simulink model "wlan_phy_rx_pmd"  (Simulink 7.8)
settings: Solver=VariableStepDiscrete  SolverMode=SingleTasking  StartTime=0.0  StopTime=rx_sim_time  RelTol=1e-3
PostLoadFcn:
  | wlan_phy_rx_init
InitFcn:
  | wlan_phy_rx_init
StartFcn:
  | wlan_phy_rx_init
values from neighbouring files (symbols this model uses):
  barker_seq20 = 0.95 * circshift(barker_seq20, [0 4]) ./ max(abs(barker_seq20))    [wlan_phy_rx_init.m]
  bit_scrambler_lfsr_bytes = sum(bit_scrambler_lfsr_bytes .* repmat(2.^[0:7], 127, 1),2)    [wlan_phy_rx_init.m]
  deinterleave_ROM = [deinterleave_ROM interleave_11n_64QAM zeros(1, 512-length(interleave_11n_64QAM))]    [wlan_phy_rx_init.m]
  longCorr_coef_i = [3*real(longCorr_coef)]    [wlan_phy_rx_init.m]
  longCorr_coef_q = [3*imag(longCorr_coef)]    [wlan_phy_rx_init.m]
  MAX_CP_LEN = 32    [wlan_phy_rx_init.m]
  MAX_NCBPS = NCBPS_11n_64QAM    (= 312)    [wlan_phy_rx_init.m]
  MAX_NUM_BYTES = 2^16-1    (= 65535)    [wlan_phy_rx_init.m]
  MAX_NUM_SC = 64    [wlan_phy_rx_init.m]
  MAX_NUM_SYMS = 600    [wlan_phy_rx_init.m]
  NCBPS_11a_BPSK = 48    [wlan_phy_rx_init.m]
  NDBPS_11a_BPSK12 = 24    [wlan_phy_rx_init.m]
  PHY_SIGNAL_MIN_LEN = 14    [wlan_phy_rx_init.m]
  preFFT_sampBuff_numSamps = 4*MAX_NUM_SC    (= 256)    [wlan_phy_rx_init.m]
  rx_sim_sig_samp_time = 8    [wlan_phy_rx_init.m]
  scr_ind_rev = zeros(1,128)    [wlan_phy_rx_init.m]
undefined symbols (not defined in the file or in its folder): gcbh, mcs_rom_11ag, mcs_rom_11n, xlBlockLoadCallback, xlGetNormalizedPeriod, xlipgetpartsetting
libraries: xbsIndex_r4 x3862, simulink x1, xbsEDKLib_r4 x1
(part 8 of 23)

== system wlan_phy_rx_pmd/CFO & Samp Buffer/Samp Buffer/S-R Latch ==
  S : Inport
  R : Inport  Port=2
  Constant1 : xbsIndex_r4/Constant  const=1  gui_display_data_type=Fixed-point  arith_type=Boolean  n_bits=1  bin_pt=0  preci_type=Single  exp_width=8  frac_width=24  explicit_period=off  period=1  equ=P=C  opselect=C  inp2=PCIN>>17  opr=+  inp1=P  carry=CIN  dbl_ovrd=off  block_type=constant
      settings: block_version=VER_STRING_GOES_HERE
  Register2 : xbsIndex_r4/Register  init=0  rst=on  en=on  dbl_ovrd=off  block_type=register
      settings: xl_area=[1 1 0 0 0 0 0]  block_version=VER_STRING_GOES_HERE
  Q : Outport
  wires:
  Constant1/1 -> Register2/1
  R/1 -> Register2/2
  Register2/1 -> Q/1
  S/1 -> Register2/3

== system wlan_phy_rx_pmd/CFO & Samp Buffer/Samp Buffer/Slice ==
  IQ : Inport
  Reinterpret2 : xbsIndex_r4/Reinterpret  force_arith_type=on  arith_type="Signed  (2's comp)"  force_bin_pt=on  bin_pt=15  block_type=reinterpret
  Reinterpret3 : xbsIndex_r4/Reinterpret  force_arith_type=on  arith_type="Signed  (2's comp)"  force_bin_pt=on  bin_pt=15  block_type=reinterpret
  Slice : xbsIndex_r4/Slice  nbits=16  boolean_output=off  mode=Upper Bit Location + Width  bit1=0  base1=MSB of Input  bit0=0  base0=LSB of Input  dbl_ovrd=off  block_type=slice
  Slice1 : xbsIndex_r4/Slice  nbits=16  boolean_output=off  mode=Lower Bit Location + Width  bit1=0  base1=MSB of Input  bit0=0  base0=LSB of Input  dbl_ovrd=off  block_type=slice
  I : Outport
  Q : Outport  Port=2
  wires:
  Slice/1 -> Reinterpret2/1
  Slice1/1 -> Reinterpret3/1
  IQ/1 -> Slice1/1, Slice/1
  Reinterpret2/1 -> I/1
  Reinterpret3/1 -> Q/1

== system wlan_phy_rx_pmd/Chan Est & EQ/Chan Est/Chan Est Offload ==
  note: These signals route to the packet buffer interface. The
      | chan ests are written to dedicated bytes in each packet
      | buffer, ahead of the packet payload. This is way
      | better than using a Sysgen shared memory block here
      | since it allows CPU High to treat the H ests as meta data
      | alongside the packet contents, with no further
      | involvement from the PHY or CPU Low.
      |
      | Estimates are written to the pkt buf after all training is
      | complete. Training from the L-LTF are written in OFDM
      | symbol 2 (SIGNAL). For 11n Rx the estimates are over-
      | written during sybmol 7 (first DATA). This assumes 1
      | spatial stream.
      |
      | The timing of CHAN_EST_VALID is critical. This signal
      | becomes the write enable on the pkt buf BRAM interface.
      | The CHAN_EST_VALID signal must *not* assert when actual
      | bytes are being written to the pkt buf. Asserting on OFDM syms
      | 2 and 7 is safe- there cannot be any decoded bits at these symbol
      | intervals. Any changes to pkt buf or decoder timing must be
      | checked for conflicts in BRAM write enable assertion between
      | chan ests and decoded bytes!
  x_ind : Inport
  x_I : Inport  Port=2
  x_Q : Inport  Port=3
  x_valid : Inport  Port=4
  sym_ind : Inport  Port=5
  Concat : xbsIndex_r4/Concat  num_inputs=2  dbl_ovrd=off  block_type=concat
  Constant1 : xbsIndex_r4/Constant  const=7  gui_display_data_type=Fixed-point  arith_type=Unsigned  n_bits=3  bin_pt=0  preci_type=Single  exp_width=8  frac_width=24  explicit_period=off  period=8  equ=P=C  opselect=C  inp2=PCIN>>17  opr=+  inp1=P  carry=CIN  dbl_ovrd=off  block_type=constant
  Constant3 : xbsIndex_r4/Constant  const=2  gui_display_data_type=Fixed-point  arith_type=Unsigned  n_bits=2  bin_pt=0  preci_type=Single  exp_width=8  frac_width=24  explicit_period=off  period=8  equ=P=C  opselect=C  inp2=PCIN>>17  opr=+  inp1=P  carry=CIN  dbl_ovrd=off  block_type=constant
  Convert1 : xbsIndex_r4/Convert  gui_display_data_type=Fixed-point  arith_type="Signed  (2's comp)"  n_bits=16  bin_pt=15  float_type=Single  exp_bits=8  fraction_bits=24  quantization=Truncate  overflow=Wrap  en=off  latency=0  dbl_ovrd=off  pipeline=off  block_type=convert
  Convert2 : xbsIndex_r4/Convert  gui_display_data_type=Fixed-point  arith_type="Signed  (2's comp)"  n_bits=16  bin_pt=15  float_type=Single  exp_bits=8  fraction_bits=24  quantization=Truncate  overflow=Wrap  en=off  latency=0  dbl_ovrd=off  pipeline=off  block_type=convert
  Logical1 : xbsIndex_r4/Logical  logical_function=OR  inputs=2  en=off  latency=0  precision=Full  arith_type=Unsigned  n_bits=16  bin_pt=0  align_bp=on  dbl_ovrd=off  block_type=logical
  Logical3 : xbsIndex_r4/Logical  logical_function=AND  inputs=2  en=off  latency=0  precision=Full  arith_type=Unsigned  n_bits=16  bin_pt=0  align_bp=on  dbl_ovrd=off  block_type=logical
  Logical4 : xbsIndex_r4/Logical  logical_function=AND  inputs=2  en=off  latency=0  precision=Full  arith_type=Unsigned  n_bits=16  bin_pt=0  align_bp=on  dbl_ovrd=off  block_type=logical
  Reinterpret : xbsIndex_r4/Reinterpret  force_arith_type=on  arith_type=Unsigned  force_bin_pt=on  bin_pt=0  block_type=reinterpret
  Reinterpret1 : xbsIndex_r4/Reinterpret  force_arith_type=on  arith_type=Unsigned  force_bin_pt=on  bin_pt=0  block_type=reinterpret
  Relational1 : xbsIndex_r4/Relational  mode=a=b  en=off  latency=0  dbl_ovrd=off  block_type=relational
  Relational3 : xbsIndex_r4/Relational  mode=a=b  en=off  latency=0  dbl_ovrd=off  block_type=relational
  tag scope: CHAN_EST_IND=global, CHAN_EST_IQ_32b=global, CHAN_EST_VALID=global
  wires:
  x_ind/1 -> [CHAN_EST_IND]
  x_I/1 -> Convert1/1
  x_Q/1 -> Convert2/1
  x_valid/1 -> Logical3/1
  sym_ind/1 -> Relational3/2, Relational1/1
  Convert2/1 -> Reinterpret1/1
  Convert1/1 -> Reinterpret/1
  Logical3/1 -> [CHAN_EST_VALID]
  Constant3/1 -> Relational3/1
  Reinterpret1/1 -> Concat/2
  Reinterpret/1 -> Concat/1
  Concat/1 -> [CHAN_EST_IQ_32b]
  Relational3/1 -> Logical1/1
  Logical1/1 -> Logical3/2
  Logical4/1 -> Logical1/2
  Constant1/1 -> Relational1/2
  Relational1/1 -> Logical4/1
  [RX_PHY_MODE_11N] -> Logical4/2

== system wlan_phy_rx_pmd/Chan Est & EQ/Chan Est/Control ==
  note: OFDM Symbols in 11a/g:
      | 0: LTF (BPSK)
      | 1: LTF (BPSK)
      | 2: SIG (BPSK)
      | 3-N: Data
  note: OFDM Symbols in 11n SISO:
      | 0: L-LTF (BPSK)
      | 1: L-LTF (BPSK)
      | 2: L-SIG (BPSK)
      | 3: HT-SIG1 (QBPSK)
      | 4: HT-SIG2 (QBPSK)
      | 5: HT-STF
      | 6: HT-LFT1
      | 7-N: Data
  note: OFDM Symbols in 11ac SISO:
      | 0: L-LTF (BPSK)
      | 1: L-LTF (BPSK)
      | 2: L-SIG (BPSK)
      | 3: VHT-SIGA1 (BPSK)
      | 4: VHT-SIGA2 (QBPSK)
      | 5: VHT-STF
      | 6: VHT-LFT1
      | 7: VHT-SIGB
      | 8-N: Data
  note: Downstream blocks should ignore Rx I/Q and H_est I/Q
      | during all training symbols in all PHY modes
  Sym Ind : Inport
  Constant1 : xbsIndex_r4/Constant  const=2  gui_display_data_type=Fixed-point  arith_type=Unsigned  n_bits=2  bin_pt=0  preci_type=Single  exp_width=8  frac_width=24  explicit_period=off  period=8  equ=P=C  opselect=C  inp2=PCIN>>17  opr=+  inp1=P  carry=CIN  dbl_ovrd=off  block_type=constant
  Constant2 : xbsIndex_r4/Constant  const=0  gui_display_data_type=Fixed-point  arith_type=Unsigned  n_bits=2  bin_pt=0  preci_type=Single  exp_width=8  frac_width=24  explicit_period=off  period=8  equ=P=C  opselect=C  inp2=PCIN>>17  opr=+  inp1=P  carry=CIN  dbl_ovrd=off  block_type=constant
  Constant3 : xbsIndex_r4/Constant  const=5  gui_display_data_type=Fixed-point  arith_type=Unsigned  n_bits=3  bin_pt=0  preci_type=Single  exp_width=8  frac_width=24  explicit_period=off  period=8  equ=P=C  opselect=C  inp2=PCIN>>17  opr=+  inp1=P  carry=CIN  dbl_ovrd=off  block_type=constant
  Constant4 : xbsIndex_r4/Constant  const=6  gui_display_data_type=Fixed-point  arith_type=Unsigned  n_bits=3  bin_pt=0  preci_type=Single  exp_width=8  frac_width=24  explicit_period=off  period=8  equ=P=C  opselect=C  inp2=PCIN>>17  opr=+  inp1=P  carry=CIN  dbl_ovrd=off  block_type=constant
  Logical1 : xbsIndex_r4/Logical  logical_function=AND  inputs=2  en=off  latency=0  precision=Full  arith_type=Unsigned  n_bits=16  bin_pt=0  align_bp=on  dbl_ovrd=off  block_type=logical
  Logical{2..3,5,7} (4 alike) : xbsIndex_r4/Logical  logical_function=OR  inputs=2  en=off  latency=0  precision=Full  arith_type=Unsigned  n_bits=16  bin_pt=0  align_bp=on  dbl_ovrd=off  block_type=logical
  Logical4 : xbsIndex_r4/Logical  logical_function=AND  inputs=2  en=off  latency=0  precision=Full  arith_type=Unsigned  n_bits=16  bin_pt=0  align_bp=on  dbl_ovrd=off  block_type=logical
  Logical6 : xbsIndex_r4/Logical  logical_function=AND  inputs=2  en=off  latency=0  precision=Full  arith_type=Unsigned  n_bits=16  bin_pt=0  align_bp=on  dbl_ovrd=off  block_type=logical
  Relational1 : xbsIndex_r4/Relational  mode=a<b  en=off  latency=0  dbl_ovrd=off  block_type=relational
  Relational{2,4..6} (4 alike) : xbsIndex_r4/Relational  mode=a=b  en=off  latency=0  dbl_ovrd=off  block_type=relational
  Relational3 : xbsIndex_r4/Relational  mode=a>=b  en=off  latency=0  dbl_ovrd=off  block_type=relational
  Est Overwrite : Outport
  Est WrEn : Outport  Port=2
  Scale : Outport  Port=3
  Sym Invalid : Outport  Port=4
  wires:
  Constant2/1 -> Relational2/1
  Constant1/1 -> Relational1/2
  Sym Ind/1 -> Relational2/2, Relational1/1, Relational4/1, Relational3/1, Relational5/1, Relational6/1
  Relational2/1 -> Logical2/1
  Relational1/1 -> Logical3/1, Logical7/1
  Logical2/1 -> Est Overwrite/1
  Logical4/1 -> Logical3/2, Logical2/2
  Logical3/1 -> Est WrEn/1
  Relational4/1 -> Logical4/1
  Constant4/1 -> Relational4/2, Relational3/2, Relational5/2
  [RX_PHY_MODE_11N_AC] -> Logical4/2, Logical1/2, Logical6/1
  Relational3/1 -> Logical1/1
  Logical1/1 -> Scale/1
  Constant3/1 -> Relational6/2
  Relational6/1 -> Logical5/1
  Relational5/1 -> Logical5/2
  Logical5/1 -> Logical6/2
  Logical7/1 -> Sym Invalid/1
  Logical6/1 -> Logical7/2

== system wlan_phy_rx_pmd/Chan Est & EQ/Chan Est/I Estimate Buffer ==
  note: data
  note: 11a/g preamble has two L-LTF. Both generate estimates and are summed
      | before the RAM. The sum must be divided by 2 to properly scale
      | the H ests for the EQ.
      | 11n/ac have 1 HT-LTF for 1SS mode, so no scaling is required.
  Est_Data : Inport
  Est_Addr : Inport  Port=2
  Est_WE : Inport  Port=3
  First Sym : Inport  Port=4
  Scale : Inport  Port=5
  AddSub2 : xbsIndex_r4/AddSub  mode=Addition  use_carryin=off  use_carryout=off  en=off  latency=0  precision=User Defined  arith_type="Signed  (2's comp)"  n_bits=18  bin_pt=15  quantization=Truncate  overflow=Flag as error  dbl_ovrd=off  use_behavioral_HDL=on  hw_selection=Fabric  pipelined=off  use_rpm=on  block_type=addsub
      settings: xl_area=[0, 0, 0, 0, 0, 0, 0]  block_version=VER_STRING_GOES_HERE
  Constant Read State : xbsIndex_r4/Constant  const=0  gui_display_data_type=Fixed-point  arith_type=Boolean  n_bits=1  bin_pt=0  preci_type=Single  exp_width=8  frac_width=24  explicit_period=off  period=1  equ=P=C  opselect=C  inp2=PCIN>>17  opr=+  inp1=P  carry=CIN  dbl_ovrd=off  block_type=constant
      settings: block_version=VER_STRING_GOES_HERE
  Constant4 : xbsIndex_r4/Constant  const=0  gui_display_data_type=Fixed-point  arith_type=Signed (2's comp)  n_bits=18  bin_pt=15  preci_type=Single  exp_width=8  frac_width=24  explicit_period=off  period=1  equ=P=C  opselect=C  inp2=PCIN>>17  opr=+  inp1=P  carry=CIN  dbl_ovrd=off  block_type=constant
      settings: block_version=VER_STRING_GOES_HERE
  Convert1 : xbsIndex_r4/Convert  gui_display_data_type=Fixed-point  arith_type="Signed  (2's comp)"  n_bits=18  bin_pt=15  float_type=Single  exp_bits=8  fraction_bits=24  quantization=Truncate  overflow=Flag as error  en=off  latency=0  dbl_ovrd=off  pipeline=off  block_type=convert
      settings: xl_area=[0, 0, 0, 0, 0, 0, 0]  block_version=VER_STRING_GOES_HERE
  Convert3 : xbsIndex_r4/Convert  gui_display_data_type=Fixed-point  arith_type="Signed  (2's comp)"  n_bits=18  bin_pt=16  float_type=Single  exp_bits=8  fraction_bits=24  quantization=Truncate  overflow=Flag as error  en=off  latency=0  dbl_ovrd=off  pipeline=off  block_type=convert
      settings: xl_area=[0, 0, 0, 0, 0, 0, 0]  block_version=VER_STRING_GOES_HERE
  Delay3 : xbsIndex_r4/Delay  rst=off  infoeditControl=Selection of Reset will increase slice count due to use of real FFs and instead of SRLs  en=off  latency=1  dbl_ovrd=off  reg_retiming=off  block_type=delay
      settings: infoedit=Hardware notes: A delay line is a chain, each link of which is an SRL16 followed by a flip-flop. If register retiming is enabled, the delay line is a chain of flip-flops.  xl_area=[0, 0, 0, 0, 0, 0, 0]  block_version=VER_STRING_GOES_HERE
  Delay6 : xbsIndex_r4/Delay  rst=off  infoeditControl=Selection of Reset will increase slice count due to use of real FFs and instead of SRLs  en=off  latency=1  dbl_ovrd=off  reg_retiming=off  block_type=delay
      settings: infoedit=Hardware notes: A delay line is a chain, each link of which is an SRL16 followed by a flip-flop. If register retiming is enabled, the delay line is a chain of flip-flops.  xl_area=[0, 0, 0, 0, 0, 0, 0]  block_version=VER_STRING_GOES_HERE
  Delay7 : xbsIndex_r4/Delay  rst=off  infoeditControl=Selection of Reset will increase slice count due to use of real FFs and instead of SRLs  en=off  latency=1  dbl_ovrd=off  reg_retiming=off  block_type=delay
      settings: infoedit=Hardware notes: A delay line is a chain, each link of which is an SRL16 followed by a flip-flop. If register retiming is enabled, the delay line is a chain of flip-flops.  xl_area=[0, 0, 0, 0, 0, 0, 0]  block_version=VER_STRING_GOES_HERE
  Dual Port RAM : xbsIndex_r4/Dual Port RAM  depth=MAX_NUM_SC  initVector=0  distributed_mem=Block RAM  init_a=0  init_b=0  rst_a=off  rst_b=on  en_a=off  en_b=off  latency=1  write_mode_A=Read After Write  write_mode_B=Read After Write  dbl_ovrd=off  optimize=Area  block_type=dpram
      settings: xl_area=[0, 0, 0, 0, 0, 0, 0]  block_version=VER_STRING_GOES_HERE
  Gateway Out{1..8} (8 alike) : xbsIndex_r4/Gateway Out  inherit_from_input=off  hdl_port=off  timing_constraint=None  locs_specified=off  LOCs={}  UseAsDAC=off  DACChannel='1'  block_type=gatewayout
  H est buff : Scope  NumInputPorts=8
      settings: TimeRange=14000  YMin=0~-0.1~-0.2~-0.4~0~0~0~-0.2  YMax=80~0.1~0.2~0.4~80~1~1~0.2  SaveName=ScopeData7
  Mux2 : xbsIndex_r4/Mux  inputs=2  en=off  latency=0  precision=Full  arith_type=Unsigned  n_bits=16  bin_pt=14  quantization=Truncate  overflow=Wrap  dbl_ovrd=off  block_type=mux
  div_2 : xbsIndex_r4/Scale  scale_factor=-1  dbl_ovrd=off  block_type=scale
  h[n] : Outport
  wires:
  Constant4/1 -> Dual Port RAM/5
  Constant Read State/1 -> Dual Port RAM/6
  AddSub2/1 -> Dual Port RAM/2, Gateway Out3/1
  Convert1/1 -> AddSub2/1
  Delay6/1 -> Dual Port RAM/1, Gateway Out8/1
  Delay3/1 -> Dual Port RAM/3, Gateway Out5/1
  Delay7/1 -> AddSub2/2, Gateway Out1/1
  Est_WE/1 -> Delay3/1
  Est_Addr/1 -> Delay6/1, Dual Port RAM/4, Gateway Out4/1
  First Sym/1 -> Dual Port RAM/7, Gateway Out6/1
  Est_Data/1 -> Delay7/1
  Dual Port RAM/2 -> Convert1/1, Gateway Out2/1
  Gateway Out8/1 -> H est buff/1  "Addr A"
  Gateway Out1/1 -> H est buff/2  "H Est"
  Gateway Out2/1 -> H est buff/3  "Port B Out"
  Gateway Out3/1 -> H est buff/4  "Port A In"
  Gateway Out4/1 -> H est buff/5  "Est Addr"
  Gateway Out5/1 -> H est buff/6  "Est We"
  Gateway Out6/1 -> H est buff/7  "Reset"
  Convert3/1 -> div_2/1, Mux2/3
  Dual Port RAM/1 -> Convert3/1, Gateway Out7/1
  Gateway Out7/1 -> H est buff/8  "Port A Out"
  Scale/1 -> Mux2/1
  div_2/1 -> Mux2/2
  Mux2/1 -> h[n]/1

== system wlan_phy_rx_pmd/Chan Est & EQ/Chan Est/Mult by LTF ==
  x_re : Inport
  x_im : Inport  Port=2
  x_ind : Inport  Port=3
  Constant1 : xbsIndex_r4/Constant  const=0  gui_display_data_type=Fixed-point  arith_type=Signed (2's comp)  n_bits=2  bin_pt=2  preci_type=Single  exp_width=8  frac_width=24  explicit_period=off  period=8  equ=P=C  opselect=C  inp2=PCIN>>17  opr=+  inp1=P  carry=CIN  dbl_ovrd=off  block_type=constant
  Constant2 : xbsIndex_r4/Constant  const=0  gui_display_data_type=Fixed-point  arith_type=Signed (2's comp)  n_bits=2  bin_pt=2  preci_type=Single  exp_width=8  frac_width=24  explicit_period=off  period=8  equ=P=C  opselect=C  inp2=PCIN>>17  opr=+  inp1=P  carry=CIN  dbl_ovrd=off  block_type=constant
  HT-LTF : xbsIndex_r4/ROM  depth=MAX_NUM_SC  initVector=ht_ltf_f  distributed_mem=Distributed memory  rst=off  init_reg=0  en=off  latency=0  gui_display_data_type=Fixed-point  arith_type="Signed  (2's comp)"  n_bits=2  bin_pt=1  preci_type=Single  exp_width=8  frac_width=24  dbl_ovrd=off  optimize=Area  use_rpm=on  block_type=sprom
  L-LTF : xbsIndex_r4/ROM  depth=MAX_NUM_SC  initVector=l_ltf_f  distributed_mem=Distributed memory  rst=off  init_reg=0  en=off  latency=0  gui_display_data_type=Fixed-point  arith_type="Signed  (2's comp)"  n_bits=2  bin_pt=1  preci_type=Single  exp_width=8  frac_width=24  dbl_ovrd=off  optimize=Area  use_rpm=on  block_type=sprom
  Mux : xbsIndex_r4/Mux  inputs=3  en=off  latency=0  precision=Full  arith_type=Unsigned  n_bits=16  bin_pt=14  quantization=Truncate  overflow=Wrap  dbl_ovrd=off  block_type=mux
  Mux1 : xbsIndex_r4/Mux  inputs=3  en=off  latency=0  precision=Full  arith_type=Unsigned  n_bits=16  bin_pt=14  quantization=Truncate  overflow=Wrap  dbl_ovrd=off  block_type=mux
  Mux2 : xbsIndex_r4/Mux  inputs=2  en=off  latency=0  precision=Full  arith_type=Unsigned  n_bits=16  bin_pt=14  quantization=Truncate  overflow=Wrap  dbl_ovrd=off  block_type=mux
  Negate : xbsIndex_r4/Negate  precision=Full  arith_type=Unsigned  n_bits=16  bin_pt=14  quantization=Truncate  overflow=Wrap  en=off  latency=0  dbl_ovrd=off  block_type=negate
  Negate1 : xbsIndex_r4/Negate  precision=Full  arith_type=Unsigned  n_bits=16  bin_pt=14  quantization=Truncate  overflow=Wrap  en=off  latency=0  dbl_ovrd=off  block_type=negate
  Reinterpret : xbsIndex_r4/Reinterpret  force_arith_type=on  arith_type=Unsigned  force_bin_pt=on  bin_pt=0  block_type=cast
      settings: block_version=VER_STRING_GOES_HERE
  H_re : Outport
  H_im : Outport  Port=2
  wires:
  Negate/1 -> Mux/4
  Constant2/1 -> Mux/2
  x_re/1 -> Mux/3, Negate/1
  x_ind/1 -> L-LTF/1, HT-LTF/1
  Mux/1 -> H_re/1
  Constant1/1 -> Mux1/2
  x_im/1 -> Negate1/1, Mux1/3
  Mux1/1 -> H_im/1
  Reinterpret/1 -> Mux/1, Mux1/1
  Negate1/1 -> Mux1/4
  [RX_PHY_MODE_11N_AC] -> Mux2/1
  L-LTF/1 -> Mux2/2
  HT-LTF/1 -> Mux2/3
  Mux2/1 -> Reinterpret/1

== system wlan_phy_rx_pmd/Chan Est & EQ/Chan Est/Q Estimate Buffer ==
  note: 11a/g preamble has two L-LTF. Both generate estimates and are summed
      | before the RAM. The sum must be divided by 2 to properly scale
      | the H ests for the EQ.
      | 11n/ac have 1 HT-LTF for 1SS mode, so no scaling is required.
  note: data
  Est_Data : Inport
  Est_Addr : Inport  Port=2
  Est_WE : Inport  Port=3
  First Sym : Inport  Port=4
  Scale : Inport  Port=5
  AddSub2 : xbsIndex_r4/AddSub  mode=Addition  use_carryin=off  use_carryout=off  en=off  latency=0  precision=User Defined  arith_type="Signed  (2's comp)"  n_bits=18  bin_pt=15  quantization=Truncate  overflow=Flag as error  dbl_ovrd=off  use_behavioral_HDL=on  hw_selection=Fabric  pipelined=off  use_rpm=on  block_type=addsub
      settings: xl_area=[0, 0, 0, 0, 0, 0, 0]  block_version=VER_STRING_GOES_HERE
  Constant Read State : xbsIndex_r4/Constant  const=0  gui_display_data_type=Fixed-point  arith_type=Boolean  n_bits=1  bin_pt=0  preci_type=Single  exp_width=8  frac_width=24  explicit_period=off  period=1  equ=P=C  opselect=C  inp2=PCIN>>17  opr=+  inp1=P  carry=CIN  dbl_ovrd=off  block_type=constant
      settings: block_version=VER_STRING_GOES_HERE
  Constant4 : xbsIndex_r4/Constant  const=0  gui_display_data_type=Fixed-point  arith_type=Signed (2's comp)  n_bits=18  bin_pt=15  preci_type=Single  exp_width=8  frac_width=24  explicit_period=off  period=1  equ=P=C  opselect=C  inp2=PCIN>>17  opr=+  inp1=P  carry=CIN  dbl_ovrd=off  block_type=constant
      settings: block_version=VER_STRING_GOES_HERE
  Convert1 : xbsIndex_r4/Convert  gui_display_data_type=Fixed-point  arith_type="Signed  (2's comp)"  n_bits=18  bin_pt=15  float_type=Single  exp_bits=8  fraction_bits=24  quantization=Truncate  overflow=Flag as error  en=off  latency=0  dbl_ovrd=off  pipeline=off  block_type=convert
      settings: xl_area=[0, 0, 0, 0, 0, 0, 0]  block_version=VER_STRING_GOES_HERE
  Convert3 : xbsIndex_r4/Convert  gui_display_data_type=Fixed-point  arith_type="Signed  (2's comp)"  n_bits=18  bin_pt=16  float_type=Single  exp_bits=8  fraction_bits=24  quantization=Truncate  overflow=Flag as error  en=off  latency=0  dbl_ovrd=off  pipeline=off  block_type=convert
      settings: xl_area=[0, 0, 0, 0, 0, 0, 0]  block_version=VER_STRING_GOES_HERE
  Delay3 : xbsIndex_r4/Delay  rst=off  infoeditControl=Selection of Reset will increase slice count due to use of real FFs and instead of SRLs  en=off  latency=1  dbl_ovrd=off  reg_retiming=off  block_type=delay
      settings: infoedit=Hardware notes: A delay line is a chain, each link of which is an SRL16 followed by a flip-flop. If register retiming is enabled, the delay line is a chain of flip-flops.  xl_area=[0, 0, 0, 0, 0, 0, 0]  block_version=VER_STRING_GOES_HERE
  Delay6 : xbsIndex_r4/Delay  rst=off  infoeditControl=Selection of Reset will increase slice count due to use of real FFs and instead of SRLs  en=off  latency=1  dbl_ovrd=off  reg_retiming=off  block_type=delay
      settings: infoedit=Hardware notes: A delay line is a chain, each link of which is an SRL16 followed by a flip-flop. If register retiming is enabled, the delay line is a chain of flip-flops.  xl_area=[0, 0, 0, 0, 0, 0, 0]  block_version=VER_STRING_GOES_HERE
  Delay7 : xbsIndex_r4/Delay  rst=off  infoeditControl=Selection of Reset will increase slice count due to use of real FFs and instead of SRLs  en=off  latency=1  dbl_ovrd=off  reg_retiming=off  block_type=delay
      settings: infoedit=Hardware notes: A delay line is a chain, each link of which is an SRL16 followed by a flip-flop. If register retiming is enabled, the delay line is a chain of flip-flops.  xl_area=[0, 0, 0, 0, 0, 0, 0]  block_version=VER_STRING_GOES_HERE
  Dual Port RAM : xbsIndex_r4/Dual Port RAM  depth=MAX_NUM_SC  initVector=0  distributed_mem=Block RAM  init_a=0  init_b=0  rst_a=off  rst_b=on  en_a=off  en_b=off  latency=1  write_mode_A=Read After Write  write_mode_B=Read After Write  dbl_ovrd=off  optimize=Area  block_type=dpram
      settings: xl_area=[0, 0, 0, 0, 0, 0, 0]  block_version=VER_STRING_GOES_HERE
  Gateway Out{1..8} (8 alike) : xbsIndex_r4/Gateway Out  inherit_from_input=off  hdl_port=off  timing_constraint=None  locs_specified=off  LOCs={}  UseAsDAC=off  DACChannel='1'  block_type=gatewayout
  Mux2 : xbsIndex_r4/Mux  inputs=2  en=off  latency=0  precision=Full  arith_type=Unsigned  n_bits=16  bin_pt=14  quantization=Truncate  overflow=Wrap  dbl_ovrd=off  block_type=mux
  div_2 : xbsIndex_r4/Scale  scale_factor=-1  dbl_ovrd=off  block_type=scale
  h[n] : Outport
  wires:
  Mux2/1 -> h[n]/1
  div_2/1 -> Mux2/2
  Scale/1 -> Mux2/1
  Gateway Out7/1 -> ?  "Port A Out"
  Dual Port RAM/1 -> Gateway Out7/1, Convert3/1
  Convert3/1 -> Mux2/3, div_2/1
  Gateway Out6/1 -> ?  "Reset"
  Gateway Out5/1 -> ?  "Est We"
  Gateway Out4/1 -> ?  "Est Addr"
  Gateway Out3/1 -> ?  "Port A In"
  Gateway Out2/1 -> ?  "Port B Out"
  Gateway Out1/1 -> ?  "H Est"
  Gateway Out8/1 -> ?  "Addr A"
  Dual Port RAM/2 -> Gateway Out2/1, Convert1/1
  Est_Data/1 -> Delay7/1
  First Sym/1 -> Gateway Out6/1, Dual Port RAM/7
  Est_Addr/1 -> Gateway Out4/1, Dual Port RAM/4, Delay6/1
  Est_WE/1 -> Delay3/1
  Delay7/1 -> Gateway Out1/1, AddSub2/2
  Delay3/1 -> Gateway Out5/1, Dual Port RAM/3
  Delay6/1 -> Gateway Out8/1, Dual Port RAM/1
  Convert1/1 -> AddSub2/1
  AddSub2/1 -> Gateway Out3/1, Dual Port RAM/2
  Constant Read State/1 -> Dual Port RAM/6
  Constant4/1 -> Dual Port RAM/5

== system wlan_phy_rx_pmd/Chan Est & EQ/Chan Est/To Float ==
  "x_I " : Inport
  "x_Q " : Inport  Port=2
  "H_I " : Inport  Port=3
  "H_Q " : Inport  Port=4
  x_ind : Inport  Port=5
  x_valid : Inport  Port=6
  sym_ind : Inport  Port=7
  Delay1 : xbsIndex_r4/Delay  rst=off  infoeditControl=Selection of Reset will increase slice count due to use of real FFs and instead of SRLs  en=off  latency=3  dbl_ovrd=off  reg_retiming=off  block_type=delay
  Delay2 : xbsIndex_r4/Delay  rst=off  infoeditControl=Selection of Reset will increase slice count due to use of real FFs and instead of SRLs  en=off  latency=3  dbl_ovrd=off  reg_retiming=off  block_type=delay
  Delay3 : xbsIndex_r4/Delay  rst=off  infoeditControl=Selection of Reset will increase slice count due to use of real FFs and instead of SRLs  en=off  latency=3  dbl_ovrd=off  reg_retiming=off  block_type=delay
  Inverter : xbsIndex_r4/Inverter  en=off  latency=0  dbl_ovrd=off  block_type=inv
  Register{,1..3} (4 alike) : xbsIndex_r4/Register  init=0  rst=on  en=off  dbl_ovrd=off  block_type=register
  To FP{1..4} (4 alike) : xbsIndex_r4/Convert  gui_display_data_type=Floating-point  arith_type=Floating-point  n_bits=16  bin_pt=14  float_type=Single  exp_bits=8  fraction_bits=24  quantization=Truncate  overflow=Wrap  en=off  latency=2  dbl_ovrd=off  pipeline=off  block_type=convert
  " x_I" : Outport
  " x_Q" : Outport  Port=2
  H_I : Outport  Port=3
  " H_Q" : Outport  Port=4
  " x_ind" : Outport  Port=5
  " x_valid" : Outport  Port=6
  " sym_ind" : Outport  Port=7
  wires:
  To FP3/1 -> " H_Q"/1
  To FP2/1 -> H_I/1
  To FP1/1 -> " x_Q"/1
  To FP4/1 -> " x_I"/1
  Register3/1 -> To FP3/1
  Register2/1 -> To FP2/1
  Inverter/1 -> Register3/2, Register2/2, Register1/2, Register/2
  Register1/1 -> To FP1/1
  Register/1 -> To FP4/1
  "x_I "/1 -> Register/1
  "x_Q "/1 -> Register1/1
  "H_I "/1 -> Register2/1
  "H_Q "/1 -> Register3/1
  x_valid/1 -> Inverter/1, Delay1/1
  Delay1/1 -> " x_valid"/1
  x_ind/1 -> Delay2/1
  Delay2/1 -> " x_ind"/1
  sym_ind/1 -> Delay3/1
  Delay3/1 -> " sym_ind"/1

== system wlan_phy_rx_pmd/Chan Est & EQ/Debug Outputs/4x Buffer ==
  I : Inport
  Q : Inport  Port=2
  Vin : Inport  Port=3
  14LSB : xbsIndex_r4/Slice  nbits=14  boolean_output=off  mode=Lower Bit Location + Width  bit1=0  base1=MSB of Input  bit0=0  base0=LSB of Input  dbl_ovrd=off  block_type=slice
  14MSB : xbsIndex_r4/Slice  nbits=14  boolean_output=off  mode=Upper Bit Location + Width  bit1=0  base1=MSB of Input  bit0=0  base0=LSB of Input  dbl_ovrd=off  block_type=slice
  Concat : xbsIndex_r4/Concat  num_inputs=2  dbl_ovrd=off  block_type=concat
  Constant3 : xbsIndex_r4/Constant  const=1  gui_display_data_type=Boolean  arith_type=Boolean  n_bits=5  bin_pt=0  preci_type=Single  exp_width=8  frac_width=24  explicit_period=on  period=4  equ=P=C  opselect=C  inp2=PCIN>>17  opr=+  inp1=P  carry=CIN  dbl_ovrd=off  block_type=constant
  FIFO : xbsIndex_r4/FIFO  mem_type=Block RAM  performance_options=Standard_FIFO  infoedit1=Available only for V4, V5, V6, V7, K7, A7 and Zynq devices.  embedded_registers=off  depth=64  percent_nbits=1  rst=off  en=off  use_dcount=off  use_percent_full_port=off  use_almost_empty=off  almost_empty_thresh=2  use_almost_full=off  almost_full_thresh=14  dbl_ovrd=off  explicit_period=off  block_type=fifo
  Reinterpret : xbsIndex_r4/Reinterpret  force_arith_type=on  arith_type=Unsigned  force_bin_pt=on  bin_pt=0  block_type=reinterpret
  Reinterpret1 : xbsIndex_r4/Reinterpret  force_arith_type=on  arith_type=Unsigned  force_bin_pt=on  bin_pt=0  block_type=reinterpret
  Reinterpret2 : xbsIndex_r4/Reinterpret  force_arith_type=on  arith_type="Signed  (2's comp)"  force_bin_pt=on  bin_pt=13  block_type=reinterpret
  Reinterpret3 : xbsIndex_r4/Reinterpret  force_arith_type=on  arith_type="Signed  (2's comp)"  force_bin_pt=on  bin_pt=13  block_type=reinterpret
  Up Sample : xbsIndex_r4/Up Sample  sample_ratio=4  copy_samples=on  en=off  latency=0  dbl_ovrd=off  block_type=usamp
  Up Sample1 : xbsIndex_r4/Up Sample  sample_ratio=4  copy_samples=on  en=off  latency=0  dbl_ovrd=off  block_type=usamp
  " I" : Outport
  " Q" : Outport  Port=2
  #Vout : Outport  Port=3
  wires:
  Constant3/1 -> FIFO/3
  Concat/1 -> FIFO/1
  I/1 -> Reinterpret/1
  14MSB/1 -> Reinterpret2/1
  Q/1 -> Reinterpret1/1
  14LSB/1 -> Reinterpret3/1
  Vin/1 -> FIFO/2
  FIFO/2 -> #Vout/1
  Reinterpret/1 -> Concat/1
  Reinterpret1/1 -> Concat/2
  Reinterpret2/1 -> Up Sample/1
  Reinterpret3/1 -> Up Sample1/1
  Up Sample/1 -> " I"/1
  Up Sample1/1 -> " Q"/1
  FIFO/1 -> 14LSB/1, 14MSB/1

== system wlan_phy_rx_pmd/Chan Est & EQ/Equalizer, Pilot Processing & PHY Mode Det/Mag ==
  I : Inport
  Q : Inport  Port=2
  AddSub : xbsIndex_r4/AddSub  mode=Addition  use_carryin=off  use_carryout=off  en=off  latency=4  precision=Full  arith_type=Unsigned  n_bits=16  bin_pt=14  quantization=Truncate  overflow=Wrap  dbl_ovrd=off  use_behavioral_HDL=off  hw_selection=DSP48  pipelined=off  block_type=addsub
  Mult : xbsIndex_r4/Mult  precision=Full  arith_type="Signed  (2's comp)"  n_bits=16  bin_pt=14  quantization=Truncate  overflow=Wrap  en=off  latency=2  dbl_ovrd=off  use_behavioral_HDL=off  opt=Speed  use_embedded=on  optimum_pipeline=off  pipeline=on  use_rpm=on  placement_style=Triangular  block_type=mult
  Mult1 : xbsIndex_r4/Mult  precision=Full  arith_type="Signed  (2's comp)"  n_bits=16  bin_pt=14  quantization=Truncate  overflow=Wrap  en=off  latency=2  dbl_ovrd=off  use_behavioral_HDL=off  opt=Speed  use_embedded=on  optimum_pipeline=off  pipeline=on  use_rpm=on  placement_style=Triangular  block_type=mult
  Register1 : xbsIndex_r4/Register  init=0  rst=off  en=off  dbl_ovrd=off  block_type=register
  Register2 : xbsIndex_r4/Register  init=0  rst=off  en=off  dbl_ovrd=off  block_type=register
  Register3 : xbsIndex_r4/Register  init=0  rst=off  en=off  dbl_ovrd=off  block_type=register
  M : Outport
  wires:
  I/1 -> Mult/1, Mult/2
  Q/1 -> Mult1/1, Mult1/2
  Mult/1 -> Register2/1
  Mult1/1 -> Register1/1
  AddSub/1 -> Register3/1
  Register2/1 -> AddSub/1
  Register1/1 -> AddSub/2
  Register3/1 -> M/1

== system wlan_phy_rx_pmd/Chan Est & EQ/Equalizer, Pilot Processing & PHY Mode Det/PHY Mode Det ==
  note: OFDM Symbols in 11a/g:
      | 0: LTF (BPSK)
      | 1: LTF (BPSK)
      | 2: SIG (BPSK)
      | 3-N: Data
  note: PHY Mode
      | 001: 11a/g
      | 010: 11n
      | 100: 11ac
  note: OFDM Symbols in 11n SISO:
      | 0: L-LTF (BPSK)
      | 1: L-LTF (BPSK)
      | 2: L-SIG (BPSK)
      | 3: HT-SIG1 (QBPSK)
      | 4: HT-SIG2 (QBPSK)
      | 5: HT-STF
      | 6: HT-LFT1
      | 7-N: Data
  note: OFDM Symbols in 11ac SISO:
      | 0: L-LTF (BPSK)
      | 1: L-LTF (BPSK)
      | 2: L-SIG (BPSK)
      | 3: VHT-SIGA1 (BPSK)
      | 4: VHT-SIGA2 (QBPSK)
      | 5: VHT-STF
      | 6: VHT-LFT1
      | 7: VHT-SIGB
      | 8-N: Data
  note: Assert rates here to avoid
      | rate ambiguity when these
      | signals are fed back to earlier
      | blocks in the pipeline
  x_I : Inport
  x_Q : Inport  Port=2
  x_valid : Inport  Port=3
  Sym ind : Inport  Port=4
  " 11ac" : xbsIndex_r4/Register  init=0  rst=on  en=on  dbl_ovrd=off  block_type=register
  " 11ag" : xbsIndex_r4/Register  init=1  rst=on  en=on  dbl_ovrd=off  block_type=register
  " 11n" : xbsIndex_r4/Register  init=0  rst=on  en=on  dbl_ovrd=off  block_type=register
  Absolute : xbsIndex_r4/Absolute  precision=User Defined  arith_type="Signed  (2's comp)"  n_bits=8  bin_pt=7  quantization=Truncate  overflow=Saturate  en=off  latency=1  dbl_ovrd=off  block_type=abs
  Absolute1 : xbsIndex_r4/Absolute  precision=User Defined  arith_type="Signed  (2's comp)"  n_bits=8  bin_pt=7  quantization=Truncate  overflow=Saturate  en=off  latency=1  dbl_ovrd=off  block_type=abs
  Assert : xbsIndex_r4/Assert  assert_type=off  type_source=Explicitly  gui_display_data_type=Fixed-point  arith_type="Signed  (2's comp)"  n_bits=16  bin_pt=14  preci_type=Single  exp_width=8  frac_width=24  assert_rate=on  rate_source=Explicitly  period=1  output_port=on  block_type=assert
  Assert1 : xbsIndex_r4/Assert  assert_type=off  type_source=Explicitly  gui_display_data_type=Fixed-point  arith_type="Signed  (2's comp)"  n_bits=16  bin_pt=14  preci_type=Single  exp_width=8  frac_width=24  assert_rate=on  rate_source=Explicitly  period=1  output_port=on  block_type=assert
  Assert2 : xbsIndex_r4/Assert  assert_type=off  type_source=Explicitly  gui_display_data_type=Fixed-point  arith_type="Signed  (2's comp)"  n_bits=16  bin_pt=14  preci_type=Single  exp_width=8  frac_width=24  assert_rate=on  rate_source=Explicitly  period=1  output_port=on  block_type=assert
  Concat : xbsIndex_r4/Concat  num_inputs=2  dbl_ovrd=off  block_type=concat
  Concat1 : xbsIndex_r4/Concat  num_inputs=3  dbl_ovrd=off  block_type=concat
  Concat2 : xbsIndex_r4/Concat  num_inputs=3  dbl_ovrd=off  block_type=concat
  Constant1 : xbsIndex_r4/Constant  const=0  gui_display_data_type=Fixed-point  arith_type=Unsigned  n_bits=2  bin_pt=0  preci_type=Single  exp_width=8  frac_width=24  explicit_period=off  period=1  equ=P=C  opselect=C  inp2=PCIN>>17  opr=+  inp1=P  carry=CIN  dbl_ovrd=off  block_type=constant
  Constant3 : xbsIndex_r4/Constant  const=3  gui_display_data_type=Fixed-point  arith_type=Unsigned  n_bits=2  bin_pt=0  preci_type=Single  exp_width=8  frac_width=24  explicit_period=off  period=1  equ=P=C  opselect=C  inp2=PCIN>>17  opr=+  inp1=P  carry=CIN  dbl_ovrd=off  block_type=constant
  Constant4 : xbsIndex_r4/Constant  const=4  gui_display_data_type=Fixed-point  arith_type=Unsigned  n_bits=3  bin_pt=0  preci_type=Single  exp_width=8  frac_width=24  explicit_period=off  period=1  equ=P=C  opselect=C  inp2=PCIN>>17  opr=+  inp1=P  carry=CIN  dbl_ovrd=off  block_type=constant
  Constant5 : xbsIndex_r4/Constant  const=1  gui_display_data_type=Fixed-point  arith_type=Unsigned  n_bits=2  bin_pt=0  preci_type=Single  exp_width=8  frac_width=24  explicit_period=off  period=1  equ=P=C  opselect=C  inp2=PCIN>>17  opr=+  inp1=P  carry=CIN  dbl_ovrd=off  block_type=constant
  Constant7 : xbsIndex_r4/Constant  const=2  gui_display_data_type=Fixed-point  arith_type=Unsigned  n_bits=2  bin_pt=0  preci_type=Single  exp_width=8  frac_width=24  explicit_period=off  period=1  equ=P=C  opselect=C  inp2=PCIN>>17  opr=+  inp1=P  carry=CIN  dbl_ovrd=off  block_type=constant
  Constant8 : xbsIndex_r4/Constant  const=2  gui_display_data_type=Fixed-point  arith_type=Unsigned  n_bits=2  bin_pt=0  preci_type=Single  exp_width=8  frac_width=24  explicit_period=off  period=1  equ=P=C  opselect=C  inp2=PCIN>>17  opr=+  inp1=P  carry=CIN  dbl_ovrd=off  block_type=constant
  Convert : xbsIndex_r4/Convert  gui_display_data_type=Fixed-point  arith_type="Signed  (2's comp)"  n_bits=9  bin_pt=7  float_type=Single  exp_bits=8  fraction_bits=24  quantization=Truncate  overflow=Wrap  en=off  latency=0  dbl_ovrd=off  pipeline=off  block_type=convert
  Convert1 : xbsIndex_r4/Convert  gui_display_data_type=Fixed-point  arith_type="Signed  (2's comp)"  n_bits=9  bin_pt=7  float_type=Single  exp_bits=8  fraction_bits=24  quantization=Truncate  overflow=Wrap  en=off  latency=0  dbl_ovrd=off  pipeline=off  block_type=convert
  Convert2 : xbsIndex_r4/Convert  gui_display_data_type=Boolean  arith_type=Boolean  n_bits=9  bin_pt=7  float_type=Single  exp_bits=8  fraction_bits=24  quantization=Truncate  overflow=Wrap  en=off  latency=0  dbl_ovrd=off  pipeline=off  block_type=convert
  Delay{1..3,5,7} (5 alike) : xbsIndex_r4/Delay  rst=off  infoeditControl=Selection of Reset will increase slice count due to use of real FFs and instead of SRLs  en=off  latency=1  dbl_ovrd=off  reg_retiming=off  block_type=delay
  Gateway Out{1..8} (8 alike) : xbsIndex_r4/Gateway Out  inherit_from_input=off  hdl_port=off  timing_constraint=None  locs_specified=off  LOCs={}  UseAsDAC=off  DACChannel='1'  block_type=gatewayout
  I_Accum : xbsIndex_r4/Accumulator  operation=Add  n_bits=16  overflow=Wrap  scale=1  rst=on  infoeditControl=reset for floating point data type must be asserted for a minimum of 2 cycles  hasbypass=off  en=on  latency=0  dbl_ovrd=off  msb_inp=100  msb=100  lsb=-100  use_behavioral_HDL=on  implementation=Fabric  block_type=accum
  LSB : xbsIndex_r4/Slice  nbits=1  boolean_output=off  mode=Lower Bit Location + Width  bit1=0  base1=MSB of Input  bit0=0  base0=LSB of Input  dbl_ovrd=off  block_type=slice
  Logical2 : xbsIndex_r4/Logical  logical_function=AND  inputs=2  en=off  latency=0  precision=Full  arith_type=Unsigned  n_bits=16  bin_pt=0  align_bp=on  dbl_ovrd=off  block_type=logical
  Logical3 : xbsIndex_r4/Logical  logical_function=OR  inputs=2  en=off  latency=0  precision=Full  arith_type=Unsigned  n_bits=16  bin_pt=0  align_bp=on  dbl_ovrd=off  block_type=logical
  Logical4 : xbsIndex_r4/Logical  logical_function=NOR  inputs=2  en=off  latency=0  precision=Full  arith_type=Unsigned  n_bits=16  bin_pt=0  align_bp=on  dbl_ovrd=off  block_type=logical
  Mod Det : Subsystem
  Mod_Sym_3 : xbsIndex_r4/Register  init=0  rst=on  en=on  dbl_ovrd=off  block_type=register
  Mod_Sym_4 : xbsIndex_r4/Register  init=0  rst=on  en=on  dbl_ovrd=off  block_type=register
  PHY Mode Det : Scope  NumInputPorts=8
      settings: TimeRange=10000  YMin=-2~-2~0~0~0~0~3.8~0.95  YMax=1.5~1.5~1~6~50~50~4.2~1.05  SaveName=ScopeData11
  Q_Accum : xbsIndex_r4/Accumulator  operation=Add  n_bits=16  overflow=Wrap  scale=1  rst=on  infoeditControl=reset for floating point data type must be asserted for a minimum of 2 cycles  hasbypass=off  en=on  latency=0  dbl_ovrd=off  msb_inp=100  msb=100  lsb=-100  use_behavioral_HDL=on  implementation=Fabric  block_type=accum
  Relational{1..4,6..7} (6 alike) : xbsIndex_r4/Relational  mode=a=b  en=off  latency=0  dbl_ovrd=off  block_type=relational
  edge : Subsystem
  negedge : Subsystem
  negedge1 : Subsystem
  11ac : Outport
  11n : Outport  Port=2
  11a/g : Outport  Port=3
  wires:
  Sym ind/1 -> Relational1/1, Relational2/1, Relational3/1, LSB/1, Gateway Out2/1
  Constant1/1 -> Relational1/2
  Relational1/1 -> Mod_Sym_3/2, Mod_Sym_4/2, " 11ag"/2, " 11n"/2, " 11ac"/2
  Constant3/1 -> Relational2/2
  Constant4/1 -> Relational3/2
  negedge/1(Q) -> Delay3/1
  Relational2/1 -> negedge/1(D)
  Convert/1 -> Absolute/1
  Relational3/1 -> negedge1/1(D)
  negedge1/1(Q) -> Delay5/1
  Convert1/1 -> Absolute1/1
  Absolute/1 -> I_Accum/1
  Absolute1/1 -> Q_Accum/1
  x_valid/1 -> Delay7/1, Gateway Out3/1
  x_I/1 -> Convert/1, Gateway Out1/1
  x_Q/1 -> Convert1/1, Gateway Out4/1
  Delay7/1 -> Q_Accum/3, I_Accum/3
  LSB/1 -> edge/1(D)
  edge/1(Q) -> Convert2/1
  I_Accum/1 -> Mod Det/1(I), Gateway Out7/1
  Q_Accum/1 -> Mod Det/2(Q), Gateway Out8/1
  Concat/1 -> Mod_Sym_3/1, Mod_Sym_4/1
  Constant5/1 -> Relational4/2
  Mod_Sym_3/1 -> Relational4/1, Relational6/1
  Constant7/1 -> Relational6/2
  Mod Det/2(QBPSK) -> Concat/1, Concat2/2
  Mod Det/3(BPSK) -> Concat/2, Concat2/3
  Mod_Sym_4/1 -> Relational7/1
  Constant8/1 -> Relational7/2
  Relational6/1 -> Logical4/2, " 11n"/1
  Relational7/1 -> Logical2/2
  Relational4/1 -> Logical2/1
  Logical2/1 -> Logical4/1, " 11ac"/1
  Logical4/1 -> " 11ag"/1
  Delay3/1 -> Mod_Sym_3/3, Delay1/1
  Delay5/1 -> Mod_Sym_4/3, Delay2/1
  Logical3/1 -> " 11ag"/3, " 11n"/3, " 11ac"/3
  " 11ag"/1 -> Concat1/3, Assert2/1
  Gateway Out1/1 -> PHY Mode Det/1  "X_I"
  Gateway Out4/1 -> PHY Mode Det/2  "X_Q"
  Gateway Out2/1 -> PHY Mode Det/4  "Sym_Ind"
  Gateway Out3/1 -> PHY Mode Det/3  "x_valid"
  Concat2/1 -> Gateway Out5/1
  Gateway Out5/1 -> PHY Mode Det/7  "mod_det"
  Gateway Out6/1 -> PHY Mode Det/8  "PHY_mode"
  Gateway Out7/1 -> PHY Mode Det/5  "I_Accum"
  Gateway Out8/1 -> PHY Mode Det/6  "Q_Accum"
  Convert2/1 -> Q_Accum/2, I_Accum/2
  Mod Det/1(QAM) -> Concat2/1
  " 11n"/1 -> Concat1/2, Assert1/1
  " 11ac"/1 -> Concat1/1, Assert/1
  Concat1/1 -> Gateway Out6/1
  Assert/1 -> 11ac/1
  Assert1/1 -> 11n/1
  Assert2/1 -> 11a/g/1
  Delay2/1 -> Logical3/1
  Delay1/1 -> Logical3/2

== system wlan_phy_rx_pmd/Chan Est & EQ/Equalizer, Pilot Processing & PHY Mode Det/Phase Err Calc ==
  CP_I : Inport
  CP_Q : Inport  Port=2
  x_valid : Inport  Port=3
  x_ind : Inport  Port=4
  sym_ind : Inport  Port=5
  Accum1 : Subsystem
  Accum2 : Subsystem
  Constant3 : xbsIndex_r4/Constant  const=0  gui_display_data_type=Fixed-point  arith_type=Unsigned  n_bits=6  bin_pt=0  preci_type=Single  exp_width=8  frac_width=24  explicit_period=off  period=8  equ=P=C  opselect=C  inp2=PCIN>>17  opr=+  inp1=P  carry=CIN  dbl_ovrd=off  block_type=constant
  Count : Subsystem
  Delay8 : xbsIndex_r4/Delay  rst=off  infoeditControl=Selection of Reset will increase slice count due to use of real FFs and instead of SRLs  en=off  latency=1  dbl_ovrd=off  reg_retiming=off  block_type=delay
  Descramble : Subsystem
  Gateway Out{3..10} (8 alike) : xbsIndex_r4/Gateway Out  inherit_from_input=off  hdl_port=off  timing_constraint=None  locs_specified=off  LOCs={}  UseAsDAC=off  DACChannel='1'  block_type=gatewayout
  Phase Calc : Subsystem
  Pilot Proc : Scope  NumInputPorts=10
      settings: TimeRange=14000  YMin=0~-0.1~-0.2~-0.4~0~0~0~-0.2~-5~-5  YMax=80~0.1~0.2~0.4~80~1~1~0.2~5~5  SaveName=ScopeData10
  Pilot Selection : Subsystem
  Register1 : xbsIndex_r4/Register  init=0  rst=off  en=on  dbl_ovrd=off  block_type=register
  Register2 : xbsIndex_r4/Register  init=0  rst=off  en=on  dbl_ovrd=off  block_type=register
  Relational1 : xbsIndex_r4/Relational  mode=a=b  en=off  latency=0  dbl_ovrd=off  block_type=relational
      settings: xl_area=[2 0 0 3 0 0 0]  block_version=VER_STRING_GOES_HERE
  Relational7 : xbsIndex_r4/Relational  mode=a=b  en=off  latency=0  dbl_ovrd=off  block_type=relational
      settings: xl_area=[2 0 0 3 0 0 0]  block_version=VER_STRING_GOES_HERE
  Sym Phase Err : Outport
  wires:
  Relational7/1 -> Count/2(Rst), Accum1/3(Rst), Accum2/3(Rst)
  Accum1/1(Sum) -> Gateway Out3/1, Phase Calc/1(I)
  Accum2/1(Sum) -> Gateway Out4/1, Phase Calc/2(Q)
  Descramble/1(" x_I") -> Accum1/2(D), Register1/1
  Descramble/2(" x_Q") -> Register2/1, Accum2/2(D)
  Delay8/1 -> Accum1/1(En), Count/1(En), Accum2/1(En), Register2/2, Register1/2
  Count/1(Last) -> Phase Calc/3(IQ Valid)
  Pilot Selection/1(Pilot) -> Delay8/1
  Pilot Selection/2(Negate) -> Descramble/1(Negate)
  CP_I/1 -> Gateway Out9/1, Descramble/2(x_I)
  CP_Q/1 -> Gateway Out10/1, Descramble/3(x_Q)
  Phase Calc/1(phase err) -> Sym Phase Err/1, Gateway Out5/1
  sym_ind/1 -> Relational1/1, Pilot Selection/3(sym_ind), Descramble/4(Sym Ind)
  Relational1/1 -> Phase Calc/4(Reset)
  x_valid/1 -> Pilot Selection/1(x_valid)
  x_ind/1 -> Pilot Selection/2(x_ind), Relational7/1, Gateway Out8/1
  Constant3/1 -> Relational7/2, Relational1/2
  Gateway Out8/1 -> Pilot Proc/1  "X Ind"
  Gateway Out3/1 -> Pilot Proc/4  "Accum I"
  Gateway Out4/1 -> Pilot Proc/5  "Accum Q"
  Gateway Out5/1 -> Pilot Proc/6  "Phase Err"
  Gateway Out6/1 -> Pilot Proc/7  "Descram I"
  Gateway Out7/1 -> Pilot Proc/8  "Descram Q"
  Gateway Out9/1 -> Pilot Proc/9  "Conj Prod I"
  Gateway Out10/1 -> Pilot Proc/10  "Conj Prod Q"
  Register1/1 -> Gateway Out6/1
  Register2/1 -> Gateway Out7/1

== system wlan_phy_rx_pmd/Chan Est & EQ/Equalizer, Pilot Processing & PHY Mode Det/a*conj(b) ==
  note: Implements A*conj(B)
  A_I : Inport
  A_Q : Inport  Port=2
  B_I : Inport  Port=3
  B_Q : Inport  Port=4
  AddSub : xbsIndex_r4/AddSub  mode=Addition  use_carryin=off  use_carryout=off  en=off  latency=4  precision=Full  arith_type=Unsigned  n_bits=16  bin_pt=14  quantization=Truncate  overflow=Wrap  dbl_ovrd=off  use_behavioral_HDL=off  hw_selection=DSP48  pipelined=off  block_type=addsub
  AddSub1 : xbsIndex_r4/AddSub  mode=Subtraction  use_carryin=off  use_carryout=off  en=off  latency=4  precision=Full  arith_type=Unsigned  n_bits=16  bin_pt=14  quantization=Truncate  overflow=Wrap  dbl_ovrd=off  use_behavioral_HDL=off  hw_selection=DSP48  pipelined=off  block_type=addsub
  Mult{1..4} (4 alike) : xbsIndex_r4/Mult  precision=Full  arith_type="Signed  (2's comp)"  n_bits=16  bin_pt=14  quantization=Truncate  overflow=Wrap  en=off  latency=2  dbl_ovrd=off  use_behavioral_HDL=off  opt=Speed  use_embedded=on  optimum_pipeline=off  pipeline=on  use_rpm=on  placement_style=Triangular  block_type=mult
  Register{1..6} (6 alike) : xbsIndex_r4/Register  init=0  rst=off  en=off  dbl_ovrd=off  block_type=register
  P_I : Outport
  P_Q : Outport  Port=2
  wires:
  A_I/1 -> Mult1/1, Mult4/2
  B_I/1 -> Mult1/2, Mult3/2
  A_Q/1 -> Mult2/1, Mult3/1
  B_Q/1 -> Mult2/2, Mult4/1
  Mult1/1 -> Register2/1
  Mult2/1 -> Register1/1
  Mult3/1 -> Register3/1
  Mult4/1 -> Register4/1
  AddSub/1 -> Register5/1
  AddSub1/1 -> Register6/1
  Register2/1 -> AddSub/1
  Register1/1 -> AddSub/2
  Register3/1 -> AddSub1/1
  Register4/1 -> AddSub1/2
  Register5/1 -> P_I/1
  Register6/1 -> P_Q/1

== system wlan_phy_rx_pmd/DSSS Rx/Demod/Bit-to-Byte ==
  Bit : Inport
  Bit Valid : Inport  Port=2
  Concat : xbsIndex_r4/Concat  num_inputs=8  dbl_ovrd=off  block_type=concat
  Constant : xbsIndex_r4/Constant  const=8  gui_display_data_type=Fixed-point  arith_type=Unsigned  n_bits=4  bin_pt=0  preci_type=Single  exp_width=8  frac_width=24  explicit_period=off  period=1  equ=P=C  opselect=C  inp2=PCIN>>17  opr=+  inp1=P  carry=CIN  dbl_ovrd=off  block_type=constant
  Counter : xbsIndex_r4/Counter  cnt_type=Free Running  cnt_to=Inf  operation=Up  start_count=0  cnt_by_val=1  arith_type=Unsigned  n_bits=8  bin_pt=0  load_pin=off  rst=on  en=on  explicit_period=off  period=1  dbl_ovrd=off  use_behavioral_HDL=off  implementation=Fabric  block_type=counter
  Counter1 : xbsIndex_r4/Counter  cnt_type=Free Running  cnt_to=Inf  operation=Up  start_count=0  cnt_by_val=1  arith_type=Unsigned  n_bits=ceil(log2(MAX_NUM_BYTES))  bin_pt=0  load_pin=off  rst=on  en=on  explicit_period=off  period=1  dbl_ovrd=off  use_behavioral_HDL=off  implementation=Fabric  block_type=counter
  Delay : xbsIndex_r4/Delay  rst=off  infoeditControl=Selection of Reset will increase slice count due to use of real FFs and instead of SRLs  en=off  latency=1  dbl_ovrd=off  reg_retiming=off  block_type=delay
  Delay1 : xbsIndex_r4/Delay  rst=off  infoeditControl=Selection of Reset will increase slice count due to use of real FFs and instead of SRLs  en=off  latency=1  dbl_ovrd=off  reg_retiming=off  block_type=delay
  Logical1 : xbsIndex_r4/Logical  logical_function=OR  inputs=2  en=off  latency=0  precision=Full  arith_type=Unsigned  n_bits=8  bin_pt=2  align_bp=on  dbl_ovrd=off  block_type=logical
      settings: xl_area=[1 0 0 1 0 0 0]  block_version=VER_STRING_GOES_HERE
  Register{,1..8} (9 alike) : xbsIndex_r4/Register  init=0  rst=on  en=on  dbl_ovrd=off  block_type=register
  Relational : xbsIndex_r4/Relational  mode=a=b  en=off  latency=0  dbl_ovrd=off  block_type=relational
  Byte : Outport
  Byte Valid : Outport  Port=2
  Byte Ind : Outport  Port=3
  wires:
  Register/1 -> Register1/1, Concat/1
  Register1/1 -> Register2/1, Concat/2
  Register2/1 -> Register3/1, Concat/3
  Register3/1 -> Register4/1, Concat/4
  Register4/1 -> Register5/1, Concat/5
  Register5/1 -> Register6/1, Concat/6
  Register6/1 -> Register7/1, Concat/7
  Bit Valid/1 -> Register1/3, Register2/3, Register3/3, Register4/3, Register5/3, Register6/3, Register7/3, Register/3, Counter/2
  [DSSS_RX_RESET] -> Register6/2, Register7/2, Register5/2, Register4/2, Register3/2, Register2/2, Register1/2, Register/2, Logical1/2
  Bit/1 -> Register/1
  Register7/1 -> Concat/8
  Concat/1 -> Register8/1
  Constant/1 -> Relational/2
  Counter/1 -> Relational/1
  Logical1/1 -> Counter/1
  Relational/1 -> Logical1/1, Register8/3, Delay1/1, Delay/1
  [DSSS_RX_RESET] -> Register8/2
  Register8/1 -> Byte/1
  Delay/1 -> Byte Valid/1
  [DSSS_RX_RESET] -> Counter1/1
  Counter1/1 -> Byte Ind/1
  Delay1/1 -> Counter1/2
